simulink model "TosAeb"  (R2021a)
settings: Solver=FixedStepDiscrete  StartTime=0.0  StopTime=inf  FixedStep=0.01  RelTol=1e-3
undefined symbols (not defined in the file or in its folder): ACCModSts, AccSts1, AEB_ObjectMovingOrientation, AEB_ObjectMovingState, CN_BTCActive, CN_CurrentGearR, CN_CurrentGearR_DHT, CN_PTCActive, CN_VDCActive, CN_VSC_EspOff, CN_VSC_FCW_AEB_Error, CN_VSC_FCW_AEB_NoDispay, CN_VSC_FCW_AEB_PerfmDgdtn, CN_VSC_FCW_AEB_TempUnAvlbl, CN_VSC_VehStandstill, CNaccelAvoidMax, CNaccelAvoidMin, CNbrakePedalPress, CNcomfortStrLeftAccelMax, CNcomfortStrLeftAccelMin, CNcomfortStrRightAccelMax, CNcomfortStrRightAccelMin, CNDrvDoorOpen, CNDrvModeEco, CNDrvModeNorm, CNDrvModeOther, CNDrvModeSport, CNDrvSBRreminder, CNNull, CNobjInvalidID, CNPassDoorOpen, CNPassSBRreminder, CNPEBSAccelNegInf, CNPEBSAccelNull, CNPEBSTimeInf, CNPEBSTimeNull, CNSTOPMAX, CNSTOPNULL, CNsubjWidth, CNSwtOFF, CNSwtON, CNTTCMAX, CNTTCNULL, CNVehConfigAEBCycNone, CNVehConfigAEBNone, CNVehConfigAEBPedNone, CNVehConfigFCWNone, CNVehConfigJANone, CollisionSeverity, FALSE, FCWSnvty, JACycObjCalcInfoDefaultParams, JAPedObjCalcInfoDefaultParams, JAVehObjCalcInfoDefaultParams, latCycObjCalcInfoDefaultParams, latPedObjCalcInfoDefaultParams, longObjCalcInfoDefaultParams, ModSts, movingStatus, ObjectType ... (+1112)
libraries: simulink x3031, AEBSFunctionLib x50, Ta_Library x33, AEBSStateMachineLib x23, Mylibrary x12, simulink_extras x7, ObjSelect_lib x3, dspstat3 x2
(part 66 of 102)

== system TosAeb/AEB_Objsel/TA_Processor/AEB_ObjSelect/TargetInfoCheck/Data_precess/TA_EffectCalculate/Obj_FilterFlgCalculate/JA_ObjFilter/CollisionProbCalc/Subsystem/PreRefPointEgoCalc ==
  subjVel : Inport  OutDataTypeStr=single
  tPredTime : Inport  Port=2  OutDataTypeStr=single
  subjLongAccel : Inport  Port=3  OutDataTypeStr=single
  subjLatAccel : Inport  Port=4  OutDataTypeStr=single
  subjYawRate : Inport  Port=5  OutDataTypeStr=single
  Add : Sum  OutDataTypeStr=single
  Gain : Gain  Gain=0.5  OutDataTypeStr=single
  Gain1 : Gain  Gain=0.5  OutDataTypeStr=single
  Math Function : Math  Operator=square  SignedPower=on  OutDataTypeStr=single  SaturateOnIntegerOverflow=on
  Product{,1..3} (4 alike) : Product  OutDataTypeStr=single
  " dxPreRefPoint" : Outport
  " dyPreRefPoint" : Outport  Port=2
  YawAnglePre : Outport  Port=3
  wires:
  Gain1/1 -> " dyPreRefPoint"/1
  Gain/1 -> Add/2
  Product3/1 -> YawAnglePre/1
  subjYawRate/1 -> Product3/2
  Product2/1 -> Gain1/1
  subjLatAccel/1 -> Product2/2
  Add/1 -> " dxPreRefPoint"/1
  subjLongAccel/1 -> Product1/2
  Math Function/1 -> Product1/1, Product2/1
  tPredTime/1 -> Product3/1, Math Function/1, Product/2
  subjVel/1 -> Product/1
  Product/1 -> Add/1
  Product1/1 -> Gain/1

== system TosAeb/AEB_Objsel/TA_Processor/AEB_ObjSelect/TargetInfoCheck/Data_precess/TA_EffectCalculate/Obj_FilterFlgCalculate/JA_ObjFilter/CollisionProbCalc/Subsystem/SubjBoundingBox ==
  dxPreRefPoint : Inport  OutDataTypeStr=single
  dyPreRefPoint : Inport  Port=2  OutDataTypeStr=single
  sincosCoef : Inport  Port=3
  Abs : Abs
  Bus Creator : BusCreator  Inputs=6
  Constant : Constant  Value=P_TA_PrmForVehRearAxleDisttoFrnt  OutDataTypeStr=single
  Constant1 : Constant  Value=P_TA_PrmForVehRearAxleDisttoRear  OutDataTypeStr=single
  Constant2 : Constant  Value=P_TA_PrmForVehWidth  OutDataTypeStr=single
  Mux : Mux
  Mux1 : Mux
  SubjLLCoordinate : Subsystem
  SubjLUCoordinate : Subsystem
  SubjRLCoordinate : Subsystem
  SubjRUCoordinate : Subsystem
  subjDxPre : Outport
  subjDyPre : Outport  Port=2
  wires:
  Constant2/1 -> Bus Creator/6  "subjWidth"
  Abs/1 -> Bus Creator/5  "dxRearBumperToRearAxis"
  Constant/1 -> Bus Creator/4  "dxFrontBumperToRearAxis"
  sincosCoef/1 -> Bus Creator/3  "sincosCoef"
  dyPreRefPoint/1 -> Bus Creator/2  "dyPreRefPoint"
  Bus Creator/1 -> SubjRLCoordinate/1(inputSignals), SubjRUCoordinate/1(inputSignals), SubjLLCoordinate/1(inputSignals), SubjLUCoordinate/1(inputSignals)
  SubjLUCoordinate/1(dxPreLU) -> [dxPreLU]  "dxPreLU"
  SubjLUCoordinate/2(dyPreLU) -> [dyPreLU]  "dyPreLU"
  SubjLLCoordinate/1(dxPreLL) -> [dxPreLL]  "dxPreLL"
  SubjLLCoordinate/2(dyPreLL) -> [dyPreLL]  "dyPreLL"
  SubjRUCoordinate/1(dxPreRU) -> [dxPreRU]  "dxPreRU"
  SubjRUCoordinate/2(dyPreRU) -> [dyPreRU]  "dyPreRU"
  SubjRLCoordinate/1(dxPreRL) -> [dxPreRL]  "dxPreRL"
  SubjRLCoordinate/2(dyPreRL) -> [dyPreRL]  "dyPreRL"
  Mux/1 -> subjDxPre/1
  Mux1/1 -> subjDyPre/1
  [dxPreRU] -> Mux/1
  [dxPreLU] -> Mux/2
  [dxPreLL] -> Mux/3
  [dxPreRL] -> Mux/4
  [dyPreRU] -> Mux1/1
  [dyPreLU] -> Mux1/2
  [dyPreLL] -> Mux1/3
  [dyPreRL] -> Mux1/4
  Constant1/1 -> Abs/1
  dxPreRefPoint/1 -> Bus Creator/1  "dxPreRefPoint"

== system TosAeb/AEB_Objsel/TA_Processor/AEB_ObjSelect/TargetInfoCheck/Data_precess/TA_EffectCalculate/Obj_FilterFlgCalculate/JA_ObjFilter/CollisionProbCalc/Subsystem/Subsystem ==
  tPredTime : Inport
  PahEstimn : Inport  Port=2  OutDataTypeStr=Bus: PahEstimn
  CalculateHeading : Subsystem
  Compare To Constant : simulink/Logic and Bit Operations/Compare To Constant  relop=<=  const=1  OutDataTypeStr=boolean
  Constant : Constant  OutDataTypeStr=uint8
  Constant1 : Constant  Value=2  OutDataTypeStr=uint8
  SegmentComponents : Subsystem
  Selector2 : Selector  IndexOptions=Index vector (port)  Indices=[1 3]  OutputSizes=1
  Switch : Switch  Criteria=u2 ~= 0
  PredHostPosnLgt : Outport
  PredHostPosnLat : Outport  Port=2
  PredHostHeading : Outport  Port=3
  wires:
  PahEstimn/1 -> Selector2/1
  [CurrentIndex] -> Selector2/2
  tPredTime/1 -> SegmentComponents/2(PredictionTime), Compare To Constant/1
  Compare To Constant/1 -> Switch/2
  Constant/1 -> Switch/1
  Switch/1 -> [CurrentIndex]
  Constant1/1 -> Switch/3
  Selector2/1 -> SegmentComponents/1(PahEstimn)  "PahEstimn"
  [dx] -> CalculateHeading/1(dx)
  [dy] -> CalculateHeading/2(dy)
  SegmentComponents/2(dx) -> [dx]
  SegmentComponents/3(PredHostPosnLat) -> PredHostPosnLat/1
  SegmentComponents/1(PredHostPosnLgt) -> PredHostPosnLgt/1
  CalculateHeading/1(PredHostHeading) -> PredHostHeading/1
  SegmentComponents/4(dy) -> [dy]

== system TosAeb/AEB_Objsel/TA_Processor/AEB_ObjSelect/TargetInfoCheck/Data_precess/TA_EffectCalculate/Obj_FilterFlgCalculate/JA_ObjFilter/CollisionProbCalc/Subsystem/ay_Filt ==
  ay : Inport
  Add : Sum  Inputs=++++
  Add1 : Sum  Inputs=++++
  Bus Creator : BusCreator  Inputs=4
  Bus Selector : BusSelector  OutputSignals=Out_ay1.ay1,Out_ay2.ay2,Out_ay3.ay3,Out_ay4.ay4
  Bus Selector1 : BusSelector  OutputSignals=Out_ay1.Count1,Out_ay2.Count2,Out_ay3.Count3,Out_ay4.Count4
  Compare To Constant : simulink/Logic and Bit Operations/Compare To Constant  relop=~=  const=0  OutDataTypeStr=boolean
  Constant : Constant  OutDataTypeStr=single
  Delay : Delay  DelayLength=1
  Delay1 : Delay
  Delay2 : Delay  DelayLength=3
  Divide : Product  Inputs=*/
  Switch : Switch  Criteria=u2 > Threshold
  ay1 : Subsystem
  ay2 : Subsystem
  ay3 : Subsystem
  ay4 : Subsystem
  ay_Filt : Outport
  wires:
  ay/1 -> Delay2/1, Delay1/1, Delay/1, ay1/1(ay)  "ay"
  Delay/1 -> ay2/1(ay)
  Delay1/1 -> ay3/1(ay)
  Delay2/1 -> ay4/1(ay)
  ay1/1(Out_ay1) -> Bus Creator/1
  ay2/1(Out_ay2) -> Bus Creator/2
  ay3/1(Out_ay3) -> Bus Creator/3
  ay4/1(Out_ay4) -> Bus Creator/4
  Bus Creator/1 -> Bus Selector1/1, Bus Selector/1
  Bus Selector/1 -> Add/1  "<ay1>"
  Bus Selector/2 -> Add/2  "<ay2>"
  Bus Selector/3 -> Add/3  "<ay3>"
  Bus Selector/4 -> Add/4  "<ay4>"
  Bus Selector1/1 -> Add1/1  "<Count1>"
  Bus Selector1/2 -> Add1/2  "<Count2>"
  Bus Selector1/3 -> Add1/3  "<Count3>"
  Bus Selector1/4 -> Add1/4  "<Count4>"
  Add1/1 -> Compare To Constant/1, Switch/1
  Compare To Constant/1 -> Switch/2
  Constant/1 -> Switch/3
  Add/1 -> Divide/1
  Switch/1 -> Divide/2
  Divide/1 -> ay_Filt/1

== system TosAeb/AEB_Objsel/TA_Processor/AEB_ObjSelect/TargetInfoCheck/Data_precess/TA_EffectCalculate/Obj_FilterFlgCalculate/JA_ObjFilter/CollisionProbCalc/Subsystem/sincosCoefCalc ==
  angle : Inport  OutDataTypeStr=single
  Bus Creator : BusCreator  Inputs=2
  Sincos : Trigonometry  Operator=sincos
  sincosCoef : Outport
  wires:
  Sincos/1 -> Bus Creator/1  "sinCoef"
  angle/1 -> Sincos/1
  Sincos/2 -> Bus Creator/2  "cosCoef"
  Bus Creator/1 -> sincosCoef/1  "sincosCoef"

== system TosAeb/AEB_Objsel/TA_Processor/AEB_ObjSelect/TargetInfoCheck/Data_precess/TA_EffectCalculate/Obj_FilterFlgCalculate/JA_ObjFilter/CollisionProbCalc/Subsystem/sincosCoefCalc1 ==
  angle : Inport  OutDataTypeStr=single
  Bus Creator : BusCreator  Inputs=2
  Sincos : Trigonometry  Operator=sincos
  sincosCoef : Outport
  wires:
  Bus Creator/1 -> sincosCoef/1  "sincosCoef"
  Sincos/2 -> Bus Creator/2  "cosCoef"
  angle/1 -> Sincos/1
  Sincos/1 -> Bus Creator/1  "sinCoef"

== system TosAeb/AEB_Objsel/TA_Processor/AEB_ObjSelect/TargetInfoCheck/Data_precess/TA_EffectCalculate/Obj_FilterFlgCalculate/JA_ObjFilter/JAObjEnable/JAObjEnableJudge/JAObjAbsVxEnable ==
  TA_ObsType : Inport
  TA_ObsVxAbs : Inport  Port=2
  Compare To Constant : simulink/Logic and Bit Operations/Compare To Constant  relop=~=  const=5  OutDataTypeStr=boolean
  Compare To Constant1 : simulink/Logic and Bit Operations/Compare To Constant  relop=~=  const=6  OutDataTypeStr=boolean
  Compare To Constant2 : simulink/Logic and Bit Operations/Compare To Constant  relop=~=  const=7  OutDataTypeStr=boolean
  Compare To Constant3 : simulink/Logic and Bit Operations/Compare To Constant  relop=<=  const=-0.5  OutDataTypeStr=boolean
  Constant : Constant  OutDataTypeStr=boolean
  Logical Operator : Logic  Inputs=3  AllPortsSameDT=off  OutDataTypeStr=boolean
  Switch : Switch  Criteria=u2 ~= 0
  JAObjAbsVxEnable : Outport
  wires:
  TA_ObsType/1 -> Compare To Constant2/1, Compare To Constant1/1, Compare To Constant/1
  Compare To Constant/1 -> Logical Operator/1
  Compare To Constant1/1 -> Logical Operator/2
  Compare To Constant2/1 -> Logical Operator/3
  Logical Operator/1 -> Switch/2
  TA_ObsVxAbs/1 -> Compare To Constant3/1
  Compare To Constant3/1 -> Switch/1
  Switch/1 -> JAObjAbsVxEnable/1
  Constant/1 -> Switch/3

== system TosAeb/AEB_Objsel/TA_Processor/AEB_ObjSelect/TargetInfoCheck/Data_precess/TA_EffectCalculate/Obj_FilterFlgCalculate/JA_ObjFilter/JAObjEnable/JAObjEnableJudge/JAObjConfEnable ==
  TA_ObsConfidence : Inport
  Compare To Constant : simulink/Logic and Bit Operations/Compare To Constant  relop=>=  const=1  OutDataTypeStr=boolean
  JAObjConfEnable : Outport
  wires:
  TA_ObsConfidence/1 -> Compare To Constant/1
  Compare To Constant/1 -> JAObjConfEnable/1

== system TosAeb/AEB_Objsel/TA_Processor/AEB_ObjSelect/TargetInfoCheck/Data_precess/TA_EffectCalculate/Obj_FilterFlgCalculate/JA_ObjFilter/JAObjEnable/JAObjEnableJudge/JAObjTypeEnable ==
  note: 0x0: Unclear
      | 0x1: Bus
      | 0x2: Car
      | 0x3: Truck
      | 0x4: Motor Bike
      | 0x5: Bicycle
      | 0x6: Pedestrian
      | 0x7: Animals
  TA_ObsType : Inport
  Compare To Constant : simulink/Logic and Bit Operations/Compare To Constant  relop=~=  const=0  OutDataTypeStr=boolean
  Compare To Constant1 : simulink/Logic and Bit Operations/Compare To Constant  relop=~=  const=7  OutDataTypeStr=boolean
  Logical Operator : Logic  AllPortsSameDT=off  OutDataTypeStr=boolean
  JAObjTypeEnable : Outport
  wires:
  TA_ObsType/1 -> Compare To Constant1/1, Compare To Constant/1
  Compare To Constant/1 -> Logical Operator/1
  Compare To Constant1/1 -> Logical Operator/2
  Logical Operator/1 -> JAObjTypeEnable/1

== system TosAeb/AEB_Objsel/TA_Processor/AEB_ObjSelect/TargetInfoCheck/Data_precess/TA_EffectCalculate/Obj_FilterFlgCalculate/Lat_ObjFilter/Lat_Objelse/Lat_ObjPre/LatObj_StateJudgment ==
  note: 状态
  Obj_Oreintation : Inport
  Obj_State : Inport  Port=2
  Obj_Vx : Inport  Port=3
  Subj_Vx : Inport  Port=4
  ObsVyAbs : Inport  Port=5
  Abs : Abs
  Abs1 : Abs
  Add : Sum
  Compare To Constant : simulink/Logic and Bit Operations/Compare To Constant  relop=~=  const=1  OutDataTypeStr=boolean
  Compare To Constant1 : simulink/Logic and Bit Operations/Compare To Constant  relop=>  const=P_TA_ObjBehaviorOnComingPara  OutDataTypeStr=boolean
  Compare To Constant2 : simulink/Logic and Bit Operations/Compare To Constant  relop=~=  const=2  OutDataTypeStr=boolean
  Compare To Constant3 : simulink/Logic and Bit Operations/Compare To Constant  relop=~=  const=3  OutDataTypeStr=boolean
  Compare To Constant4 : simulink/Logic and Bit Operations/Compare To Constant  relop=>=  const=P_TA_ObjBehaviorLatStationPara  OutDataTypeStr=boolean
  Compare To Constant5 : simulink/Logic and Bit Operations/Compare To Constant  relop=~=  const=6  OutDataTypeStr=boolean
  Compare To Constant6 : simulink/Logic and Bit Operations/Compare To Constant  relop===  const=3  OutDataTypeStr=boolean
  Compare To Constant7 : simulink/Logic and Bit Operations/Compare To Constant  relop===  const=2  OutDataTypeStr=boolean
  Compare To Constant8 : simulink/Logic and Bit Operations/Compare To Constant  relop=>=  const=0.78  OutDataTypeStr=boolean
  Logical Operator : Logic  Operator=OR  Inputs=3  AllPortsSameDT=off  OutDataTypeStr=boolean
  Logical Operator1 : Logic  AllPortsSameDT=off  OutDataTypeStr=boolean
  Logical Operator2 : Logic  Operator=OR  AllPortsSameDT=off  OutDataTypeStr=boolean
  Logical Operator3 : Logic  Operator=OR  Inputs=3  AllPortsSameDT=off  OutDataTypeStr=boolean
  Logical Operator4 : Logic  AllPortsSameDT=off  OutDataTypeStr=boolean
  Logical Operator5 : Logic  Inputs=3  AllPortsSameDT=off  OutDataTypeStr=boolean
  Relational Operator : RelationalOperator  Operator=<=  OutDataTypeStr=boolean  RndMeth=Simplest
  Sin : Trigonometry  Operator=atan2
  State_Enable : Outport
  wires:
  Obj_Oreintation/1 -> Compare To Constant5/1, Compare To Constant/1, Compare To Constant6/1, Compare To Constant7/1
  Obj_Vx/1 -> Add/1
  Subj_Vx/1 -> Add/2
  Add/1 -> Abs/1, Compare To Constant1/1
  Compare To Constant/1 -> Logical Operator/1
  ObsVyAbs/1 -> Abs1/1
  Obj_State/1 -> Compare To Constant3/1, Compare To Constant2/1
  Compare To Constant1/1 -> Logical Operator/3
  Abs/1 -> [abs_vx], Relational Operator/1
  Compare To Constant4/1 -> Logical Operator1/2
  Compare To Constant2/1 -> Logical Operator2/1
  Compare To Constant3/1 -> Logical Operator2/2
  Abs1/1 -> Relational Operator/2, [abs_vy], Compare To Constant4/1
  Logical Operator1/1 -> Logical Operator4/1
  Logical Operator2/1 -> Logical Operator4/2
  Sin/1 -> Compare To Constant8/1
  Compare To Constant8/1 -> Logical Operator3/1
  Compare To Constant7/1 -> Logical Operator3/2
  Compare To Constant6/1 -> Logical Operator3/3
  Logical Operator/1 -> Logical Operator5/2
  Logical Operator3/1 -> Logical Operator5/1
  Logical Operator4/1 -> Logical Operator5/3
  Relational Operator/1 -> Logical Operator1/1
  Compare To Constant5/1 -> Logical Operator/2
  [abs_vy] -> Sin/1
  [abs_vx] -> Sin/2
  Logical Operator5/1 -> State_Enable/1

== system TosAeb/AEB_Objsel/TA_Processor/AEB_ObjSelect/TargetInfoCheck/Data_precess/TA_EffectCalculate/Obj_FilterFlgCalculate/Lat_ObjFilter/Lat_Objelse/Lat_ObjPre/LatObj_TypeJudgment ==
  Obj_Type : Inport
  Compare To Constant : simulink/Logic and Bit Operations/Compare To Constant  relop===  const=4  OutDataTypeStr=boolean
  Compare To Constant1 : simulink/Logic and Bit Operations/Compare To Constant  relop===  const=5  OutDataTypeStr=boolean
  Compare To Constant2 : simulink/Logic and Bit Operations/Compare To Constant  relop===  const=6  OutDataTypeStr=boolean
  Logical Operator : Logic  Operator=OR  Inputs=3  AllPortsSameDT=off  OutDataTypeStr=boolean
  Type_Enable : Outport
  wires:
  Obj_Type/1 -> Compare To Constant2/1, Compare To Constant1/1, Compare To Constant/1
  Compare To Constant/1 -> Logical Operator/1
  Compare To Constant1/1 -> Logical Operator/2
  Compare To Constant2/1 -> Logical Operator/3
  Logical Operator/1 -> Type_Enable/1

== system TosAeb/AEB_Objsel/TA_Processor/AEB_ObjSelect/TargetInfoCheck/Data_precess/TA_EffectCalculate/Obj_FilterFlgCalculate/Lat_ObjFilter/Lat_Objelse/Lat_ObjPre/Subj_State ==
  Subj_Vx : Inport
  YawRate : Inport  Port=2
  Add : Sum
  Compare To Constant : simulink/Logic and Bit Operations/Compare To Constant  relop=>  const=P_TA_PrmForVehSpdStationary  OutDataTypeStr=boolean
  Constant : Constant  Value=P_TA_PrmForVehRearAxleDisttoFrnt
  Product : Product
  Product1 : Product
  Product2 : Product
  Sqrt : Sqrt  SaturateOnIntegerOverflow=on
  Subj_State : Outport
  wires:
  Subj_Vx/1 -> Product/2, Product/1
  YawRate/1 -> Product1/1
  Constant/1 -> Product1/2
  Product1/1 -> Product2/2, Product2/1
  Product/1 -> Add/1
  Product2/1 -> Add/2
  Add/1 -> Sqrt/1
  Sqrt/1 -> Compare To Constant/1
  Compare To Constant/1 -> Subj_State/1

== system TosAeb/AEB_Objsel/TA_Processor/AEB_ObjSelect/TargetInfoCheck/Data_precess/TA_EffectCalculate/Obj_FilterFlgCalculate/Lat_ObjFilter/Lat_Objelse/Lat_ObjPre/Subsystem ==
  note: 现阶段判断：dy > 0 处于车辆的右侧
      |                       dy < 0 处于车辆的左侧
      | 目标由左到右横穿：vy > 0
      | 目标由右到左横穿：vy < 0
  dx : Inport
  dy : Inport  Port=2
  ax : Inport  Port=3
  ay : Inport  Port=4
  Width : Inport  Port=5
  Oreintation : Inport  Port=6
  vx : Inport  Port=7
  vy : Inport  Port=8
  LatDis_Cal : Subsystem
  LatDis_Cal1 : Subsystem  Commented=on
  Lat_ObjIntersect : Outport
  Lat_TTR : Outport  Port=2
  wires:
  dy/1 -> LatDis_Cal/1(dy)
  Width/1 -> LatDis_Cal/2(Width)
  vy/1 -> LatDis_Cal/4(vy)
  Oreintation/1 -> LatDis_Cal/3(Oreintation)
  LatDis_Cal/2(Lat_TTR) -> Lat_TTR/1
  LatDis_Cal/1(Lat_ObjIntersect) -> Lat_ObjIntersect/1
  dx/1 -> LatDis_Cal/5(dx)
  ax/1 -> LatDis_Cal/6(ax)
  vx/1 -> LatDis_Cal/8(vx)
  ay/1 -> LatDis_Cal/7(ay)

== system TosAeb/AEB_Objsel/TA_Processor/AEB_ObjSelect/TargetInfoCheck/Data_precess/TA_EffectCalculate/Obj_FilterFlgCalculate/Lat_ObjFilter/Lat_Objelse/Lat_ObjPre/Type_State ==
  Type_Enable : Inport
  State_Enable : Inport  Port=2
  Subj_State : Inport  Port=3
  Logical Operator : Logic  Inputs=3  AllPortsSameDT=off  OutDataTypeStr=boolean
  LatObj_Valid : Outport
  wires:
  Type_Enable/1 -> Logical Operator/1
  State_Enable/1 -> Logical Operator/2
  Logical Operator/1 -> LatObj_Valid/1
  Subj_State/1 -> Logical Operator/3

== system TosAeb/AEB_Objsel/TA_Processor/AEB_ObjSelect/TargetInfoCheck/Data_precess/TA_EffectCalculate/Obj_FilterFlgCalculate/Long_ObjFilter/Info_Calculate/ObjWeight_Jugement/Subsystem ==
  Obj_Vy : Inport  OutDataTypeStr=single
  Obj_Vx : Inport  Port=2
  Subj_Vx : Inport  Port=3
  Subj_Yaw : Inport  Port=4
  State : Inport  Port=5
  AEB_State : Inport  Port=6
  Obj_HeadAg : Inport  Port=7
  Abs : Abs
  Abs1 : Abs
  Abs2 : Abs
  Add : Sum
  Add1 : Sum
  Add2 : Sum
  Compare To Constant : simulink/Logic and Bit Operations/Compare To Constant  relop===  const=3  OutDataTypeStr=boolean
  Compare To Constant1 : simulink/Logic and Bit Operations/Compare To Constant  relop=<=  const=2.36  OutDataTypeStr=boolean
  Compare To Constant2 : simulink/Logic and Bit Operations/Compare To Constant  relop=>=  const=0.78  OutDataTypeStr=boolean
  Compare To Constant3 : simulink/Logic and Bit Operations/Compare To Constant  relop===  const=2  OutDataTypeStr=boolean
  Constant : Constant  Value=P_TA_PrmForVehRearAxleDisttoFrnt
  Constant1 : Constant  Value=0  OutDataTypeStr=boolean
  Constant2 : Constant  Value=0.5  OutDataTypeStr=single
  Logical Operator1 : Logic  Operator=OR  AllPortsSameDT=off  OutDataTypeStr=boolean
  Logical Operator2 : Logic  AllPortsSameDT=off  OutDataTypeStr=boolean
  Logical Operator3 : Logic  Operator=OR  AllPortsSameDT=off  OutDataTypeStr=boolean
  Product : Product
  Relational Operator : RelationalOperator  OutDataTypeStr=boolean  RndMeth=Simplest
  Switch : Switch  Criteria=u2 > Threshold
  Switch1 : Switch  Criteria=u2 ~= 0
  Unit Delay : UnitDelay  SampleTime=-1  HasFrameUpgradeWarning=on
  Spd_Valid : Outport
  wires:
  Subj_Vx/1 -> Add1/2
  Obj_Vy/1 -> Add/1
  Add1/1 -> Abs1/1
  Add/1 -> Abs/1
  Obj_HeadAg/1 -> Abs2/1
  Abs2/1 -> Compare To Constant2/1, Compare To Constant1/1
  Subj_Yaw/1 -> Product/1
  Relational Operator/1 -> Logical Operator1/1
  Obj_Vx/1 -> Add1/1
  [Subj_Vy] -> Add/2
  Logical Operator2/1 -> Logical Operator1/2
  Product/1 -> [Subj_Vy]
  Constant/1 -> Product/2
  Abs/1 -> Relational Operator/1
  Compare To Constant2/1 -> Logical Operator2/1
  Compare To Constant1/1 -> Logical Operator2/2
  State/1 -> Compare To Constant3/1, Compare To Constant/1
  Compare To Constant3/1 -> Logical Operator3/1
  Compare To Constant/1 -> Logical Operator3/2
  Logical Operator3/1 -> Switch/2
  Constant1/1 -> Switch/1
  Abs1/1 -> Add2/1
  Add2/1 -> Relational Operator/2
  Constant2/1 -> Add2/2
  AEB_State/1 -> Switch1/2
  Switch/1 -> Switch1/3
  Unit Delay/1 -> Switch1/1
  Logical Operator1/1 -> Switch/3
  Switch1/1 -> Spd_Valid/1, Unit Delay/1

== system TosAeb/AEB_Objsel/TA_Processor/AEB_ObjSelect/TargetInfoCheck/Data_precess/TA_EffectCalculate/Obj_FilterFlgCalculate/Long_ObjFilter/OverlapCalculate/BoudingBoxCalculate_Else/Overlap_FlgCalculate ==
  PosnLgtHost : Inport
  PosnLatHost : Inport  Port=2
  HeadingHost : Inport  Port=3
  Obj_LongPosn : Inport  Port=4
  Obj_LatPosn : Inport  Port=5
  Obj_PreHeading : Inport  Port=6
  Relative_LongPosn : Inport  Port=7
  Relative_LatPosn : Inport  Port=8
  Obj_Length : Inport  Port=9
  Obj_Width : Inport  Port=10
  AEB_State : Inport  Port=11
  AbsPosn_InsectionFlg : Subsystem
  OverlapFlagJudgement : Subsystem
  RelativePosn_InsectionFlg : Subsystem
  Boudingbox_Calculate : Outport
  wires:
  Relative_LongPosn/1 -> RelativePosn_InsectionFlg/1(Relative_LongPosn)
  Relative_LatPosn/1 -> RelativePosn_InsectionFlg/2(Relative_LatPosn)
  [Obj_Length] -> RelativePosn_InsectionFlg/4(Obj_Length)
  [Obj_Width] -> RelativePosn_InsectionFlg/5(Obj_Width)
  PosnLgtHost/1 -> AbsPosn_InsectionFlg/1(PosnLgtHost)
  PosnLatHost/1 -> [PosnLatHost], AbsPosn_InsectionFlg/2(PosnLatHost)
  HeadingHost/1 -> AbsPosn_InsectionFlg/3(HeadingHost)
  Obj_LongPosn/1 -> AbsPosn_InsectionFlg/4(Obj_LongPosn)
  Obj_LatPosn/1 -> [Obj_LatPosn], AbsPosn_InsectionFlg/5(Obj_LatPosn)
  Obj_PreHeading/1 -> AbsPosn_InsectionFlg/6(Obj_PreHeading)
  Obj_Length/1 -> [Obj_Length], AbsPosn_InsectionFlg/7(Obj_Length)
  Obj_Width/1 -> [Obj_Width], AbsPosn_InsectionFlg/8(Obj_Width)
  AbsPosn_InsectionFlg/1(Abs_OverlapFlg) -> OverlapFlagJudgement/1(Abs_OverlapFlg)
  RelativePosn_InsectionFlg/1(Relative_OverlapFlg) -> OverlapFlagJudgement/2(Relative_OverlapFlg)
  OverlapFlagJudgement/1(Boudingbox_Calculate) -> Boudingbox_Calculate/1
  AEB_State/1 -> RelativePosn_InsectionFlg/3(AEB_State)
  [Obj_LatPosn] -> RelativePosn_InsectionFlg/6(Obj_LatPosn)
  [PosnLatHost] -> RelativePosn_InsectionFlg/7(PosnLatHost)

== system TosAeb/AEB_Objsel/TA_Processor/AEB_ObjSelect/TargetInfoCheck/Data_precess/TA_EffectCalculate/Obj_FilterFlgCalculate/Long_ObjFilter/OverlapCalculate/BoudingBoxCalculate_Else/PosnatTTI ==
  TimeToReachLgt : Inport
  PahEstimn : Inport  Port=2
  TA_ObjInfo : Inport  Port=3
  VehSelfData : Inport  Port=4
  Bus Selector : BusSelector  OutputSignals=TA_ObsLength,TA_ObsWidth
  Bus Selector1 : BusSelector  OutputSignals=VehSelf.AEB_Intervention_ESP
  LongitudinalMotionData : Subsystem
  PredictHostPositionAtTTR : Subsystem
  PredictObjectPositionAtTTR : Subsystem
  PredictRelativePositionAtTTR : Subsystem
  PosnLgtHost : Outport
  PosnLatHost : Outport  Port=2
  HeadingHost : Outport  Port=3
  Obj_LongPosn : Outport  Port=4
  Obj_LatPosn : Outport  Port=5
  Obj_PreHeading : Outport  Port=6
  Relative_LongPosn : Outport  Port=7
  Relative_LatPosn : Outport  Port=8
  Obj_Length : Outport  Port=9
  Obj_Width : Outport  Port=10
  AEB_State : Outport  Port=11
  wires:
  LongitudinalMotionData/5(Obj_dx) -> PredictObjectPositionAtTTR/6(Obj_dx), [Obj_dx]
  LongitudinalMotionData/10(Ref_ay) -> PredictRelativePositionAtTTR/4(Ref_ay)
  TA_ObjInfo/1 -> LongitudinalMotionData/1(TA_ObjInfo), [TA_ObjInfo]
  LongitudinalMotionData/2(Obj_Vy) -> PredictObjectPositionAtTTR/3(Obj_Vy)
  [Obj_dx] -> PredictRelativePositionAtTTR/5(Obj_dx)
  LongitudinalMotionData/6(Obj_dy) -> [Obj_dy], PredictObjectPositionAtTTR/7(Obj_dy)
  [Obj_dy] -> PredictRelativePositionAtTTR/6(Obj_dy)
  LongitudinalMotionData/8(Ref_Vy) -> PredictRelativePositionAtTTR/2(Ref_Vy)
  TimeToReachLgt/1 -> [TTR], PredictHostPositionAtTTR/1(PredictionTime)
  LongitudinalMotionData/1(Obj_Vx) -> PredictObjectPositionAtTTR/2(Obj_Vx)
  LongitudinalMotionData/3(Obj_Ax) -> PredictObjectPositionAtTTR/4(Obj_Ax)
  PahEstimn/1 -> PredictHostPositionAtTTR/2(PahEstimn)
  LongitudinalMotionData/7(Ref_Vx) -> PredictRelativePositionAtTTR/1(Ref_Vx)
  [TTR] -> PredictRelativePositionAtTTR/7(ttr)
  [TTR] -> PredictObjectPositionAtTTR/1(ttr)
  PredictRelativePositionAtTTR/2(Relative_LongPosn) -> Relative_LongPosn/1
  LongitudinalMotionData/4(Obj_Ay) -> PredictObjectPositionAtTTR/5(Obj_Ay)
  LongitudinalMotionData/9(Ref_ax) -> PredictRelativePositionAtTTR/3(Ref_ax)
  PredictHostPositionAtTTR/1(PredHostPosnLgt) -> PosnLgtHost/1  "PosnLgtHost"
  PredictHostPositionAtTTR/2(PredHostPosnLat) -> PosnLatHost/1  "PosnLatHost"
  PredictRelativePositionAtTTR/3(Relative_LatPosn) -> Relative_LatPosn/1
  PredictHostPositionAtTTR/3(PredHostHeading) -> HeadingHost/1  "HeadingHost"
  PredictObjectPositionAtTTR/1(Obj_LongPosn) -> Obj_LongPosn/1
  PredictObjectPositionAtTTR/2(Obj_LatPosn) -> Obj_LatPosn/1
  [TA_ObjInfo] -> Bus Selector/1
  PredictObjectPositionAtTTR/3(Obj_PreHeading) -> Obj_PreHeading/1
  VehSelfData/1 -> [VehSelfData], PredictHostPositionAtTTR/3(VehSelfData)
  [VehSelfData] -> PredictRelativePositionAtTTR/8(VehSelfData)
  Bus Selector/1 -> Obj_Length/1  "<TA_ObsLength>"
  Bus Selector/2 -> Obj_Width/1  "<TA_ObsWidth>"
  [VehSelfData] -> Bus Selector1/1
  Bus Selector1/1 -> AEB_State/1  "<AEB_Intervention_ESP>"
  PredictRelativePositionAtTTR/1(TTCtemp) -> PredictObjectPositionAtTTR/8(TTCtemp)

== system TosAeb/AEB_Objsel/TA_Processor/AEB_ObjSelect/TargetInfoCheck/Data_precess/TA_EffectCalculate/Obj_FilterFlgCalculate/Long_ObjFilter/TTR_Calculate/CalculateTTRAndTTM_else/LongitudinalTTRComputer ==
  MotionDataFrontClosest : Inport
  MotionDataRearClosest : Inport  Port=2
  StoppingData : Inport  Port=3
  EdgeDistanceFrontClosest : Inport  Port=4
  EdgeDistanceRearClosest : Inport  Port=5
  PosnLgt : Inport  Port=6
  VehSelfData : Inport  Port=7
  Bus Selector : BusSelector  OutputSignals=VRel
  CalculateTTI : Subsystem  TreatAsAtomicUnit=on
  DetermineLongitudinalClosestSide : Subsystem
  FindWhichMotionDataToUse : Subsystem
  SelectTTI : Subsystem
  TimeToIntersection : Outport
  SolutionExists : Outport  Port=2
  wires:
  CalculateTTI/3(StationaryObjectTTI) -> SelectTTI/3(StationaryObjectTTI)
  CalculateTTI/2(StationaryHostTTI) -> SelectTTI/2(StationaryHostTTI)
  Bus Selector/1 -> DetermineLongitudinalClosestSide/2(VLgtRel)  "<VRel>"
  CalculateTTI/1(MovingTTI) -> SelectTTI/1(MovingTTI)
  FindWhichMotionDataToUse/4(EdgeDistanceAlternativeSetup) -> CalculateTTI/4(EdgeDistanceAlternativeSetup)
  FindWhichMotionDataToUse/3(EdgeDistanceNormalSetup) -> CalculateTTI/3(EdgeDistanceNormalSetup)
  FindWhichMotionDataToUse/1(MotionDataNormalSetup) -> CalculateTTI/1(MotionDataNormalSetup)
  FindWhichMotionDataToUse/2(MotionDataAlternativeSetup) -> CalculateTTI/2(MotionDataAlternativeSetup)
  EdgeDistanceRearClosest/1 -> FindWhichMotionDataToUse/5(EdgeDistanceRearClosest)  "EdgeDistanceRearClosest"
  EdgeDistanceFrontClosest/1 -> FindWhichMotionDataToUse/4(EdgeDistanceFrontClosest)  "EdgeDistanceFrontClosest"
  MotionDataRearClosest/1 -> FindWhichMotionDataToUse/3(MotionDataRearClosest)  "MotionDataRearClosest"
  DetermineLongitudinalClosestSide/1(HostFrontIsClosest) -> FindWhichMotionDataToUse/1(HostFrontIsClosest)
  PosnLgt/1 -> DetermineLongitudinalClosestSide/1(PosnLgt)  "PosnLgt"
  SelectTTI/1(TimeToIntersection) -> TimeToIntersection/1
  MotionDataFrontClosest/1 -> Bus Selector/1, FindWhichMotionDataToUse/2(MotionDataFrontClosest)  "MotionDataFrontClosest"
  CalculateTTI/4(MovingSolutionExists) -> SelectTTI/4(MovingSolutionExists)
  CalculateTTI/5(StationaryHostSolutionExists) -> SelectTTI/5(StationaryHostSolutionExists)
  CalculateTTI/6(StationaryObjectSolutionExists) -> SelectTTI/6(StationaryObjectSolutionExists)
  StoppingData/1 -> SelectTTI/7(StoppingData), CalculateTTI/6(StoppingData)  "StoppingData"
  SelectTTI/2(SolutionExists) -> SolutionExists/1
  VehSelfData/1 -> CalculateTTI/5(VehSelfData)

== system TosAeb/AEB_Objsel/TA_Processor/AEB_ObjSelect/TargetInfoCheck/Data_precess/TA_EffectCalculate/Obj_FilterFlgCalculate/Long_ObjFilter/TTR_Calculate/CalculateTTRAndTTM_else/ModifyTTRAtSameSpeedAndAcceleration ==
  note: Avoid floating-point equality.
  CloseEdgeDistance : Inport
  FarEdgeDistance : Inport  Port=2
  VRel : Inport  Port=3
  ARel : Inport  Port=4
  TimeToReachIn : Inport  Port=5
  Abs : Abs
  Compare To Zero : Subsystem  mask(Compare To Zero){relop=<, OutDataTypeStr=boolean, ZeroCross=on}
      mask prompts: relop "Simulink:masks:Operator_MP"; OutDataTypeStr "Simulink:masks:OutputDataType_MP"; ZeroCross "Simulink:masks:EnableZerocrossingDetection_MP"
      mask help: Simulink:masks:CompareToZero_MD
      mask init:
      | blkName = gcb;
      | 
      | if strcmp(get_param(gcbh,'OutDataTypeStr'),'boolean')
      | 	setValue = 'boolean';
      | else
      |     setValue = 'fixdt(0, 8)';
      | end
      | 
      | set_param([blkName,'/Compare'],'relop',relop, ...
      | 	'OutDataTypeStr', setValue, 'ZeroCross', ZeroCross);
      mask TypeOptions relop: == | ~= | < | <= | >= | >
      mask TypeOptions OutDataTypeStr: Simulink:masks:uint8 | Simulink:masks:boolean
      mask Callback ZeroCross: sl('zeroCrossEnable', gcbh);
      mask Display: disp([relop,' 0'])
  Compare To Zero1 : Subsystem  mask(Compare To Zero){relop=<, OutDataTypeStr=boolean, ZeroCross=on}
      mask prompts: relop "Simulink:masks:Operator_MP"; OutDataTypeStr "Simulink:masks:OutputDataType_MP"; ZeroCross "Simulink:masks:EnableZerocrossingDetection_MP"
      mask help: Simulink:masks:CompareToZero_MD
      mask init: same as TosAeb/AEB_Objsel/TA_Processor/AEB_ObjSelect/TargetInfoCheck/Data_precess/TA_EffectCalculate/Obj_FilterFlgCalculate/Long_ObjFilter/TTR_Calculate/CalculateTTRAndTTM_else/ModifyTTRAtSameSpeedAndAcceleration/Compare To Zero
      mask TypeOptions relop: == | ~= | < | <= | >= | >
      mask TypeOptions OutDataTypeStr: Simulink:masks:uint8 | Simulink:masks:boolean
      mask Callback ZeroCross: sl('zeroCrossEnable', gcbh);
      mask Display: disp([relop,' 0'])
  Logical Operator : Logic  Operator=XOR  AllPortsSameDT=off  OutDataTypeStr=boolean
  Logical Operator1 : Logic  AllPortsSameDT=off  OutDataTypeStr=boolean
  Saturation_1 : Saturate  UpperLimit=P_TA_TTIUpperLimiPositive  LowerLimit=P_TA_TTILowerLimitNegative
      settings: AttributesFormatString=Limits:\n[%<LowerLimit>,\n%<UpperLimit>]
  Saturation_2 : Saturate  UpperLimit=P_TA_TTIUpperLimiPositive  LowerLimit=P_TA_TTILowerLimitNegative
      settings: AttributesFormatString=Limits:\n[%<LowerLimit>,\n%<UpperLimit>]
  SizeChecker : Subsystem  mask(Size checker){UseInput=off, Limit=1e-2, DataTypeIn=single, Operator=<}
      mask prompts: UseInput "Take the limit as an input"; DataTypeIn "Data type:"; Operator "Relational operator:"
      mask help: The block tests the absolute value of the supplied signal against a specified value using the selected operator. If the signal conforms to the test, true is returned; else, false is returned. The limit can either be set in the mask or as an external signal.
      mask init:
      | % Author: Jakob Andersson (jande573)
      | 
      | %% Prepare the block switch
      | % Define what block to replace
      | oldBlockPath=[gcb '/LimitSource'];
      | 
      | % Get block settings to preserve
      | annotation=get_param(oldBlockPath,'AttributesFormatString');
      | position=get_param(oldBlockPath,'Position');
      | orientation=get_param(oldBlockPath,'Orientation');
      | dt=get_param(oldBlockPath,'OutDataTypeStr'); % Data type (make sure there is no name conflict with the mask parameter DataType)
      | 
      | %% Check what type of switch to perform and execute it
      | switch UseInput
      |     case 'on'
      |         % Define the replacement
      |         newBlock='built-in/Inport';
      |         
      |         % Replace the block (if necessary)
      |         if(~strcmp('Inport',get_param(oldBlockPath,'BlockType')))
      |             delete_block(oldBlockPath);
      |             add_block(newBlock,oldBlockPath,'AttributesFormatString',annotation,'Position',position,'Orientation',orientation,'OutDataTypeStr','Inherit: auto');
      |         end
      |     case 'off'
      |         % Define the replacement
      |         newBlock='built-in/Constant';
      |         
      |         % Replace the block (if necessary)
      |         if(~strcmp('Constant',get_param(oldBlockPath,'BlockType')))
      |             delete_block(oldBlockPath);
      |             add_block(newBlock,oldBlockPath,'AttributesFormatString',annotation,'Position',position,'Orientation',orientation,'OutDataTypeStr','Inherit: Inherit via back propagation');
      |         
      |             % Assign the value from the mask to the new block
      |             set_param(oldBlockPath,'Value','Limit');
      |         end
      | end
      settings: AttributesFormatString=DT=%<DataTypeIn>
      mask Help: The block tests the absolute value of the supplied signal against a specified value using the selected operator. If the signal conforms to the test, true is returned; else, false is returned. The limit can either be set in the mask or as an external signal.
      mask Callback UseInput:
      | % Author: Jakob Andersson (jande573)
      | 
      | % Enable and disable the box for the limit variable depending on the status of the checkbox
      | if strcmp(get_param(gcb,'UseInput'),'on')
      |     % Disable the box
      |     set_param(gcb,'MaskEnables',{'on','off','on','on'}); % One setting for each parameter in this mask; needs to be updated if the parameters are changed
      | else
      |     % Enable the box
      |     set_param(gcb,'MaskEnables',{'on','on','on','on'}); % One setting for each parameter in this mask; needs to be updated if the parameters are changed
      | end
      mask Callback Limit:
      | % Author: Jakob Andersson (jande573)
      | 
      | % Define the default value to apply if the entered value is disallowed
      | default='1e-6';
      | 
      | % Evaluate the entered value
      | val=eval(get_param(gcb,'Limit'));
      | 
      | % Test the entered value and revert to the default if necessary
      | if isnumeric(val)==false
      |     % Set the default if the entered value did not evaluate to a numeric
      |     set_param(gcb,'Limit',default);
      |     errordlg('The limit must be numeric. The default value will be used instead.');
      | elseif val<0
      |     % Set the default if the entered value is negative
      |     set_param(gcb,'Limit',default);
      |     errordlg('The limit must be positive since it is compared to an absolute value. The default value will be used instead.');
      | end
      mask TypeOptions Operator:
      | Relational
      | Operator/Operator
      mask Callback Operator:
      | % Author: Jakob Andersson (jande573)
      | 
      | % Define the default value to apply if the entered value is disallowed
      | EnabledOperators={'==','~=','<','<=','>=','>'};
      | default='>';
      | 
      | % Evaluate the selected operator and determine if it is ok
      | ok=any(strcmp(get_param(gcb,'Operator'),EnabledOperators));
      | 
      | % Revert the operator if it is disabled
      | if ~ok
      |     % Set the default if the entered value did not evaluate to a numeric
      |     set_param(gcb,'Operator',default);
      |     errordlg('The selected operator is disabled. The default operator will be used instead.');
      | end
      mask Display:
      | % Display the mask for this block
      | % Author: Jakob Andersson (jande573)
      | 
      | % Transform the operator to TeX
      | switch Operator
      |     case '=='
      |         op='=';
      |     case '~='
      |         op='\neq';
      |     case '<='
      |         op='\leq';
      |     case '>='
      |         op='\geq';
      |     otherwise
      |         op=Operator;
      | end
      | 
      | % Display the correct mask depending on the number of inputs
      | if strcmp(UseInput,'on')
      |     % Display the block logic and the set limit
      |     disp(horzcat('|u| ',op,' a'),'texmode','on');
      | 
      |     % Display the port title
      |     port_label('input',1,'u');
      |     port_label('input',2,'a');
      | else
      |     % Display the block logic and the set limit
      |     disp(horzcat('|u| ',op,' ',num2str(Limit)),'texmode','on');
      | 
      |     % Display the port title
      |     port_label('input',1,'u');
      | end
  SizeChecker1 : Subsystem  mask(Size checker){UseInput=off, Limit=1e-2, DataTypeIn=single, Operator=<}
      mask prompts: UseInput "Take the limit as an input"; DataTypeIn "Data type:"; Operator "Relational operator:"
      mask help: The block tests the absolute value of the supplied signal against a specified value using the selected operator. If the signal conforms to the test, true is returned; else, false is returned. The limit can either be set in the mask or as an external signal.
      mask init: same as TosAeb/AEB_Objsel/TA_Processor/AEB_ObjSelect/TargetInfoCheck/Data_precess/TA_EffectCalculate/Obj_FilterFlgCalculate/Long_ObjFilter/TTR_Calculate/CalculateTTRAndTTM_else/ModifyTTRAtSameSpeedAndAcceleration/SizeChecker
      settings: AttributesFormatString=DT=%<DataTypeIn>
      mask Help: The block tests the absolute value of the supplied signal against a specified value using the selected operator. If the signal conforms to the test, true is returned; else, false is returned. The limit can either be set in the mask or as an external signal.
      mask Callback UseInput:
      | % Author: Jakob Andersson (jande573)
      | 
      | % Enable and disable the box for the limit variable depending on the status of the checkbox
      | if strcmp(get_param(gcb,'UseInput'),'on')
      |     % Disable the box
      |     set_param(gcb,'MaskEnables',{'on','off','on','on'}); % One setting for each parameter in this mask; needs to be updated if the parameters are changed
      | else
      |     % Enable the box
      |     set_param(gcb,'MaskEnables',{'on','on','on','on'}); % One setting for each parameter in this mask; needs to be updated if the parameters are changed
      | end
      mask Callback Limit:
      | % Author: Jakob Andersson (jande573)
      | 
      | % Define the default value to apply if the entered value is disallowed
      | default='1e-6';
      | 
      | % Evaluate the entered value
      | val=eval(get_param(gcb,'Limit'));
      | 
      | % Test the entered value and revert to the default if necessary
      | if isnumeric(val)==false
      |     % Set the default if the entered value did not evaluate to a numeric
      |     set_param(gcb,'Limit',default);
      |     errordlg('The limit must be numeric. The default value will be used instead.');
      | elseif val<0
      |     % Set the default if the entered value is negative
      |     set_param(gcb,'Limit',default);
      |     errordlg('The limit must be positive since it is compared to an absolute value. The default value will be used instead.');
      | end
      mask TypeOptions Operator:
      | Relational
      | Operator/Operator
      mask Callback Operator:
      | % Author: Jakob Andersson (jande573)
      | 
      | % Define the default value to apply if the entered value is disallowed
      | EnabledOperators={'==','~=','<','<=','>=','>'};
      | default='>';
      | 
      | % Evaluate the selected operator and determine if it is ok
      | ok=any(strcmp(get_param(gcb,'Operator'),EnabledOperators));
      | 
      | % Revert the operator if it is disabled
      | if ~ok
      |     % Set the default if the entered value did not evaluate to a numeric
      |     set_param(gcb,'Operator',default);
      |     errordlg('The selected operator is disabled. The default operator will be used instead.');
      | end
      mask Display:
      | % Display the mask for this block
      | % Author: Jakob Andersson (jande573)
      | 
      | % Transform the operator to TeX
      | switch Operator
      |     case '=='
      |         op='=';
      |     case '~='
      |         op='\neq';
      |     case '<='
      |         op='\leq';
      |     case '>='
      |         op='\geq';
      |     otherwise
      |         op=Operator;
      | end
      | 
      | % Display the correct mask depending on the number of inputs
      | if strcmp(UseInput,'on')
      |     % Display the block logic and the set limit
      |     disp(horzcat('|u| ',op,' a'),'texmode','on');
      | 
      |     % Display the port title
      |     port_label('input',1,'u');
      |     port_label('input',2,'a');
      | else
      |     % Display the block logic and the set limit
      |     disp(horzcat('|u| ',op,' ',num2str(Limit)),'texmode','on');
      | 
      |     % Display the port title
      |     port_label('input',1,'u');
      | end
  Switch : Switch  Criteria=u2 ~= 0
  Switch1 : Switch  Criteria=u2 ~= 0
  TiUpprLimIn : Constant  Value=P_TA_TTILowerLimitNegative
  TiUpprLimIn1 : Constant  Value=P_TA_TTIUpperLimiPositive
  Valid_Time : Outport
  TimeToReach : Outport  Port=2
  wires:
  Logical Operator/1 -> Switch1/2  "Overlap"
  Compare To Zero1/1(y) -> Logical Operator/2  "NegativeFarEdgeDistance"
  Compare To Zero/1(y) -> Logical Operator/1  "NegativeCloseEdgeDistance"
  FarEdgeDistance/1 -> Compare To Zero1/1(u)  "FarEdgeDistance"
  CloseEdgeDistance/1 -> Compare To Zero/1(u)  "CloseEdgeDistance"
  Logical Operator1/1 -> Switch/2  "ModifyTTR"
  Switch/1 -> Saturation_2/1, Saturation_1/1
  TiUpprLimIn/1 -> Switch1/1
  TimeToReachIn/1 -> Switch/3  "TimeToReachIn"
  SizeChecker/1(TestOk) -> Logical Operator1/1  "SameSpeed"
  SizeChecker1/1(TestOk) -> Logical Operator1/2  "SameAcceleration"
  VRel/1 -> SizeChecker/1(Signal)  "VRel"
  ARel/1 -> SizeChecker1/1(Signal)  "ARel"
  Switch1/1 -> Switch/1  "DefaultTTR"
  TiUpprLimIn1/1 -> Switch1/3
  Saturation_1/1 -> TimeToReach/1
  Abs/1 -> Valid_Time/1
  Saturation_2/1 -> Abs/1

== system TosAeb/AEB_Objsel/TA_Processor/AEB_ObjSelect/TargetInfoCheck/Data_precess/TA_EffectCalculate/Obj_FilterFlgCalculate/Long_ObjFilter/TTR_Calculate/CalculateTTRAndTTM_else/Obj_AbsInfo ==
  TA_ObjInfo : Inport
  VehSelfData : Inport  Port=2
  InforForTTICalculation : Subsystem
  LongitudinalEdgeToEdgeDistances : Subsystem
  LongitudinalMotionData : Subsystem
  StoppingDataForTTICalculation : Subsystem
  MotionDataFrontClosest : Outport
  MotionDataRearClosest : Outport  Port=2
  StoppingData : Outport  Port=3
  CloseEdgeDistanceFrontClosest : Outport  Port=4
  CloseEdgeDistanceRearClosest : Outport  Port=5
  FarEdgeDistanceFrontClosest : Outport  Port=6
  wires:
  TA_ObjInfo/1 -> [TA_ObjInfo], LongitudinalMotionData/1(TA_ObjInfo)
  VehSelfData/1 -> LongitudinalMotionData/2(VehSelfData)
  LongitudinalMotionData/1(Obj_RefVx) -> InforForTTICalculation/1(VRel)
  LongitudinalMotionData/2(Obj_AbsVx) -> [Obj_AbsVx], InforForTTICalculation/2(VLgtObj)
  LongitudinalMotionData/3(Subj_Vx) -> [Subj_AbsVx], InforForTTICalculation/3(VLgtHost)
  LongitudinalMotionData/4(Obj_RefAcclx) -> InforForTTICalculation/4(ARel)
  LongitudinalMotionData/5(Obj_AbsAcclx) -> [Obj_AbsAcclx], InforForTTICalculation/5(ALgtObj)
  LongitudinalMotionData/6(Subj_Ax) -> [Subj_AbsAx], InforForTTICalculation/6(ALgtHost)
  InforForTTICalculation/2(MotionDataRearClosest) -> MotionDataRearClosest/1
  InforForTTICalculation/1(MotionDataFrontClosest) -> MotionDataFrontClosest/1
  [Obj_AbsVx] -> StoppingDataForTTICalculation/1(VLgtObj)
  [Obj_AbsAcclx] -> StoppingDataForTTICalculation/2(ALgtObj)
  [Subj_AbsVx] -> StoppingDataForTTICalculation/3(VLgtHost)
  [Subj_AbsAx] -> StoppingDataForTTICalculation/4(ALgtHost)
  [TA_ObjInfo] -> StoppingDataForTTICalculation/5(TA_ObjInfo)
  StoppingDataForTTICalculation/1(StoppingData) -> StoppingData/1
  [TA_ObjInfo] -> LongitudinalEdgeToEdgeDistances/1(TA_ObjInfo)
  LongitudinalEdgeToEdgeDistances/1(CloseEdgeDistanceFrontClosest) -> CloseEdgeDistanceFrontClosest/1
  LongitudinalEdgeToEdgeDistances/2(CloseEdgeDistanceRearClosest) -> CloseEdgeDistanceRearClosest/1
  LongitudinalEdgeToEdgeDistances/3(FarEdgeDistanceFrontClosest) -> FarEdgeDistanceFrontClosest/1

== system TosAeb/AEB_Objsel/TA_Processor/AEB_ObjSelect/TargetInfoCheck/Data_precess/TA_EffectCalculate/Obj_FilterFlgCalculate/Long_ObjFilter/TTR_Calculate/CalculateTTRAndTTM_else/SetCorrectSign ==
  note: 100
  note: -100
  TTI_in : Inport
  SolutionExists : Inport  Port=2
  CloseEdgeDistance : Inport  Port=3
  FarEdgeDistance : Inport  Port=4
  Compare To Zero1 : Subsystem  mask(Compare To Zero){relop=<, OutDataTypeStr=boolean, ZeroCross=on}
      mask prompts: relop "Simulink:masks:Operator_MP"; OutDataTypeStr "Simulink:masks:OutputDataType_MP"; ZeroCross "Simulink:masks:EnableZerocrossingDetection_MP"
      mask help: Simulink:masks:CompareToZero_MD
      mask init: same as TosAeb/AEB_Objsel/TA_Processor/AEB_ObjSelect/TargetInfoCheck/Data_precess/TA_EffectCalculate/Obj_FilterFlgCalculate/Long_ObjFilter/TTR_Calculate/CalculateTTRAndTTM_else/ModifyTTRAtSameSpeedAndAcceleration/Compare To Zero
      mask TypeOptions relop: == | ~= | < | <= | >= | >
      mask TypeOptions OutDataTypeStr: Simulink:masks:uint8 | Simulink:masks:boolean
      mask Callback ZeroCross: sl('zeroCrossEnable', gcbh);
      mask Display: disp([relop,' 0'])
  Compare To Zero2 : Subsystem  mask(Compare To Zero){relop=<, OutDataTypeStr=boolean, ZeroCross=on}
      mask prompts: relop "Simulink:masks:Operator_MP"; OutDataTypeStr "Simulink:masks:OutputDataType_MP"; ZeroCross "Simulink:masks:EnableZerocrossingDetection_MP"
      mask help: Simulink:masks:CompareToZero_MD
      mask init: same as TosAeb/AEB_Objsel/TA_Processor/AEB_ObjSelect/TargetInfoCheck/Data_precess/TA_EffectCalculate/Obj_FilterFlgCalculate/Long_ObjFilter/TTR_Calculate/CalculateTTRAndTTM_else/ModifyTTRAtSameSpeedAndAcceleration/Compare To Zero
      mask TypeOptions relop: == | ~= | < | <= | >= | >
      mask TypeOptions OutDataTypeStr: Simulink:masks:uint8 | Simulink:masks:boolean
      mask Callback ZeroCross: sl('zeroCrossEnable', gcbh);
      mask Display: disp([relop,' 0'])
  Logical Operator : Logic  Operator=XOR  AllPortsSameDT=off  OutDataTypeStr=boolean
  Switch1 : Switch  Criteria=u2 ~= 0
  Switch2 : Switch  Criteria=u2 ~= 0
  TiUpprLimIn1 : Constant  Value=P_TA_TTILowerLimitNegative
  TiUpprLimIn2 : Constant  Value=P_TA_TTIUpperLimiPositive
  TTI_out : Outport
  wires:
  Logical Operator/1 -> Switch1/2
  CloseEdgeDistance/1 -> Compare To Zero1/1(u)  "CloseEdgeDistance"
  FarEdgeDistance/1 -> Compare To Zero2/1(u)  "FarEdgeDistance"
  Compare To Zero1/1(y) -> Logical Operator/1  "NegativeCloseEdgeDistance"
  Compare To Zero2/1(y) -> Logical Operator/2  "NegativeFarEdgeDistance"
  Switch2/1 -> TTI_out/1
  TTI_in/1 -> Switch2/1
  Switch1/1 -> Switch2/3
  TiUpprLimIn1/1 -> Switch1/1
  TiUpprLimIn2/1 -> Switch1/3
  SolutionExists/1 -> Switch2/2

== system TosAeb/AEB_Objsel/TA_Processor/AEB_ObjSelect/TargetInfoCheck/Data_precess/TA_EffectCalculate/Obj_FilterFlgCalculate/Long_ObjFilter/TTR_Calculate/CalculateTTRAndTTM_else/Subsystem ==
  Long_ObjWeightCoef : Inport
  TA_ObjInfo : Inport  Port=2
  VehSelfData : Inport  Port=3
  TimeToReach : Inport  Port=4
  Bus Selector : BusSelector  OutputSignals=TA_ObsDx  OutputAsBus=on
  Bus Selector1 : BusSelector  OutputSignals=VehSelf.ABS_VehicleSpeed
  Chart1 : Stateflow chart  Commented=on  TreatAsAtomicUnit=on
  Compare To Constant : simulink/Logic and Bit Operations/Compare To Constant  relop=>=  const=P_TA_TTIValidNumber  OutDataTypeStr=boolean
  Compare To Constant1 : simulink/Logic and Bit Operations/Compare To Constant  relop===  const=255  OutDataTypeStr=boolean
  Compare To Constant2 : simulink/Logic and Bit Operations/Compare To Constant  relop=<  const=0.1  OutDataTypeStr=boolean
  Compare To Constant3 : simulink/Logic and Bit Operations/Compare To Constant  relop=>=  const=P_TA_TimegapThred  OutDataTypeStr=boolean
  Constant : Constant  Value=255  OutDataTypeStr=uint8
  Constant1 : Constant  Value=P_TA_TTIValidNumber
  Constant2 : Constant  Value=0.1  OutDataTypeStr=single
  Divide : Product  Inputs=*/
  Logical Operator : Logic  AllPortsSameDT=off  OutDataTypeStr=boolean
  Logical Operator1 : Logic  AllPortsSameDT=off  OutDataTypeStr=boolean
  Logical Operator2 : Logic  Operator=NOT  AllPortsSameDT=off  OutDataTypeStr=boolean
  Switch : Switch  Criteria=u2 ~= 0
  Switch1 : Switch  Criteria=u2 > Threshold
  Long_ObjWeightCoefTTI : Outport
  wires:
  Long_ObjWeightCoef/1 -> Compare To Constant1/1, Switch/1
  Switch/1 -> Long_ObjWeightCoefTTI/1
  TimeToReach/1 -> Compare To Constant/1
  Constant/1 -> Switch/3
  Constant1/1 -> Chart1/3(P_TA_TTIValidNumber)
  Compare To Constant1/1 -> Logical Operator/1
  Logical Operator/1 -> Logical Operator2/1
  TA_ObjInfo/1 -> Bus Selector/1
  VehSelfData/1 -> Bus Selector1/1
  Bus Selector1/1 -> Switch1/3, Compare To Constant2/1  "<ABS_VehicleSpeed>"
  Compare To Constant2/1 -> Switch1/2
  Constant2/1 -> Switch1/1
  Bus Selector/1 -> Divide/1
  Switch1/1 -> Divide/2
  Divide/1 -> Compare To Constant3/1
  Compare To Constant3/1 -> Logical Operator1/1
  Compare To Constant/1 -> Logical Operator1/2
  Logical Operator1/1 -> Logical Operator/2
  Logical Operator2/1 -> Switch/2
